simulink library "LibrarySimulinkSASv2016a"  (R2018a)
settings: Solver=ode45  StartTime=0.0  StopTime=10.0  RelTol=1e-3
undefined symbols (not defined in the file or in its folder): IdB, IdL, IdM, shmControl, ts
libraries: LibrarySimulinkSAS x15, $bdroot x1
(part 3 of 4)

== system LibrarySimulinkSASv2016a/V-REP Camera ==
  "Position " : Inport
  Orientation : Inport  Port=2
  Generic Share Memory Block V2.0 : S-Function  FunctionName=matlabGenericSHM  Parameters=nombre,dispositivo,vecE,vecS,tipoE,tipoS,ts  SFunctionDeploymentMode=off  EnableBusSupport=off  mask(Generic Share Memory block V2.0){nombre='vrepCam', dispositivo=id, vecE=[3 3], vecS=[3*ResX*ResY], tipoE=['f' 'f'], tipoS=['C'], ts=-1}
      mask prompts: nombre "Share Memory base name"; dispositivo "Device Number"; vecE "Sizes input vector"; vecS "Sizes output vector"; tipoE "Data Types Inputs"; tipoS "Data Types Outputs"; ts "Sample Time"
      mask help:
      | This block allows Simulink communication with external processes through shared memory. It works on Unix and Windows.
      | More information in Help.
      mask Help:
      | Share Memory base name (nombre): It's the base string of share memory ej 'robot'
      | Device Number (dispositivo): integer that identifies the device between multiple in use
      | Two shared memorys are created with names:
      | "%name_entrada_%dispositivo" for the device input (writing)
      | "%name_salida_%dispositivo" for the device output (reading)
      | Sizes input vector (vecE)  : array that specify the number of inputs (array dimension) and the inputs size (array values)
      | Sizes output vector  (vecS): array that specify the number of outputs (array dimension) and the outputs size (array values)
      | Data types input(tipoE): char array that specify each input types
      | Data types output (tipoS): char array that specify each output types
      | Allowed types are:
      | ('f'=float, 'd'=double, 'i'=int32, 'c'=char, 'C'= unsigned char, 'I'=unsigned int)
      | Example.
      | Suppose the following parameters:
      | nombre='exampleMemory'
      | dispositivo=10
      | vecE=[3 2 1]
      | vecS=[6 5 7 8]
      | tipoE=['f' 'I' 'c']
      | tipoS=['C' 'i' 'd' 'd']
      | 
      | Two Share Memories are created with names:
      | "exampleMemory_entrada_10" (block input)
      | "exampleMemory_salida_10"  (block output)
      | 3 inputs are created:
      |  1° Dimension 3, type "unsigned char"
      |  2° Dimension 2, type "int"
      |  3° Dimension 1, type "char"
      | 4 outputs are created:
      |  1° Dimension 6, type "float"
      |  2° Dimension 5, type "unsigned int"
      |  3° Dimension 7, type "double"
      |  3° Dimension 8, type "double"
      mask Display: image('shmem.jpg')
  toRGB image1 : Subsystem  mask{ResX=ResX, ResY=ResY}
      mask prompts: ResX "Resolucion Camara X"; ResY "Resolucion Camara Y"
      mask Display:
      | port_label('output', 1, 'RGB')
      | port_label('input', 1, 'videoIN')
  RGB : Outport
  charBuff : Outport  Port=2
  wires:
  "Position "/1 -> Generic Share Memory Block V2.0/1
  toRGB image1/1(Out1) -> RGB/1
  Orientation/1 -> Generic Share Memory Block V2.0/2
  Generic Share Memory Block V2.0/1 -> toRGB image1/1(In1), charBuff/1

== system LibrarySimulinkSASv2016a/V-REP Display ==
  "RGB " : Inport
  Generic Shared Memory Block V2 : LibrarySimulinkSAS/Generic Shared Memory Block V2  devInMem='device_actions'  devOutMem='device_sensors'  vecE=[3*ResX*ResY]  vecS=[]  tipoE=['C']  tipoS=[]  ts=ts
  Permute Dimensions : PermuteDimensions  Order=[3,2,1]
  Reshape : Reshape
  toSingle : DataTypeConversion  OutDataTypeStr=uint8
  wires:
  Permute Dimensions/1 -> Reshape/1
  Reshape/1 -> Generic Shared Memory Block V2/1
  "RGB "/1 -> toSingle/1
  toSingle/1 -> Permute Dimensions/1

== system LibrarySimulinkSASv2016a/V-REP Fast Dynamic Joint ==
  jointControl : Inport
  controlType : Inport  Port=2
  PID : Inport  Port=3
  Data Type Conversion : DataTypeConversion
  Data Type Conversion1 : DataTypeConversion
  Data Type Conversion2 : DataTypeConversion
  Generic Shared Memory Block : LibrarySimulinkSAS/Generic Shared Memory Block  nombre='memoriaEjemplo'  dispositivo=10  vecE=[3 2 1]  vecS=[4 5 6 156]  tipoE=['f' 'I' 'c']  tipoS=['C' 'i' 'd' 'd']  ts=0
  cnv : DataTypeConversion
  cnv1 : DataTypeConversion
  cnv2 : DataTypeConversion
  position : Outport
  velocity : Outport  Port=2
  acceleration : Outport  Port=3
  wires:
  Data Type Conversion2/1 -> Generic Shared Memory Block/3
  Data Type Conversion1/1 -> Generic Shared Memory Block/2
  Data Type Conversion/1 -> Generic Shared Memory Block/1
  cnv1/1 -> velocity/1
  cnv/1 -> position/1
  Generic Shared Memory Block/1 -> cnv/1
  Generic Shared Memory Block/2 -> cnv1/1
  controlType/1 -> Data Type Conversion1/1
  jointControl/1 -> Data Type Conversion/1
  cnv2/1 -> acceleration/1
  Generic Shared Memory Block/3 -> cnv2/1
  PID/1 -> Data Type Conversion2/1

== system LibrarySimulinkSASv2016a/V-REP GyroSensor ==
  Data Type Conversion : DataTypeConversion
  Simple Share Memory Block : S-Function  FunctionName=simpleSHM  Parameters=nombre,dispositivo,vecE,vecS,tipoE,tipoS  SFunctionDeploymentMode=off  EnableBusSupport=off  mask(Share Memory Block version 2.0){nombre='VREP_gyroData', dispositivo=n, vecE=[], vecS=[3], tipoE=[], tipoS=['f']}
      mask prompts: nombre "Share Memory base name"; dispositivo "Device Number"; vecE "Sizes input vector"; vecS "Sizes output vector"; tipoE "Data Types Inputs"; tipoS "Data Types Outputs"
      mask help: This block allows Simulink communication with external processes through shared memory. It works on Unix and Windows
      mask Help:
      | Share Memory base name (nombre): It's the base string of share memory ej 'robot'
      | Device Number (dispositivo): integer that identifies the device between multiple in use
      | Only one Share memory will be created, so you should specify only the inputs or outputs but not both
      | One shared memorys is created with name: "%name_%dispositivo"
      | 
      | Sizes input vector (vecE)  : array that specify the number of inputs (array dimension) and the inputs size (array values)
      | Sizes output vector  (vecS): array that specify the number of outputs (array dimension) and the outputs size (array values)
      | Data types input(tipoE): char array that specify each input types
      | Data types output (tipoS): char array that specify each output types
      | Allowed types are:
      | ('f'=float, 'd'=double, 'i'=int32, 'c'=char, 'C'= unsigned char, 'I'=unsigned int)
      | 
      | Example.
      | Suppose the following parameters:
      | nombre='exampleMemory'
      | dispositivo=10
      | vecE=[3 2 1]
      | vecS=[]
      | tipoE=['f' 'I' 'c']
      | tipoS=[]
      | 
      | One Share Memorie is created with name:
      | "exampleMemory_10" (block input)
      | 
      | 3 inputs are created:
      |  1° Dimension 3, type "unsigned char"
      |  2° Dimension 2, type "int"
      |  3° Dimension 1, type "char"
      mask Display: image('memoryChip.png')
  GyroData : Outport
  wires:
  Data Type Conversion/1 -> GyroData/1
  Simple Share Memory Block/1 -> Data Type Conversion/1

== system LibrarySimulinkSASv2016a/V-REP Hokuyo ==
  Generic Shared Memory Block V2.0 : S-Function  FunctionName=matlabGenericSHM  Parameters=nombre,dispositivo,vecE,vecS,tipoE,tipoS,ts  SFunctionDeploymentMode=off  EnableBusSupport=off  mask(Generic Share Memory block V2.0){nombre='vrepHOKUYO', dispositivo=id, vecE=[], vecS=[1 1 1]*683, tipoE=[], tipoS=['f' 'f' 'f'], ts=ts}
      mask prompts: nombre "Share Memory base name"; dispositivo "Device Number"; vecE "Sizes input vector"; vecS "Sizes output vector"; tipoE "Data Types Inputs"; tipoS "Data Types Outputs"; ts "Sample Time"
      mask help:
      | This block allows Simulink communication with external processes through shared memory. It works on Unix and Windows.
      | More information in Help.
      mask Help:
      | Share Memory base name (nombre): It's the base string of share memory ej 'robot'
      | Device Number (dispositivo): integer that identifies the device between multiple in use
      | Two shared memorys are created with names:
      | "%name_entrada_%dispositivo" for the device input (writing)
      | "%name_salida_%dispositivo" for the device output (reading)
      | Sizes input vector (vecE)  : array that specify the number of inputs (array dimension) and the inputs size (array values)
      | Sizes output vector  (vecS): array that specify the number of outputs (array dimension) and the outputs size (array values)
      | Data types input(tipoE): char array that specify each input types
      | Data types output (tipoS): char array that specify each output types
      | Allowed types are:
      | ('f'=float, 'd'=double, 'i'=int32, 'c'=char, 'C'= unsigned char, 'I'=unsigned int)
      | Example.
      | Suppose the following parameters:
      | nombre='exampleMemory'
      | dispositivo=10
      | vecE=[3 2 1]
      | vecS=[6 5 7 8]
      | tipoE=['f' 'I' 'c']
      | tipoS=['C' 'i' 'd' 'd']
      | 
      | Two Share Memories are created with names:
      | "exampleMemory_entrada_10" (block input)
      | "exampleMemory_salida_10"  (block output)
      | 3 inputs are created:
      |  1° Dimension 3, type "unsigned char"
      |  2° Dimension 2, type "int"
      |  3° Dimension 1, type "char"
      | 4 outputs are created:
      |  1° Dimension 6, type "float"
      |  2° Dimension 5, type "unsigned int"
      |  3° Dimension 7, type "double"
      |  3° Dimension 8, type "double"
      mask Display: image('shmem.jpg')
  a doble : DataTypeConversion  OutDataTypeStr=double
  a doble1 : DataTypeConversion  OutDataTypeStr=double
  a doble3 : DataTypeConversion  OutDataTypeStr=double
  ang (rad) : Outport
  ang (deg) : Outport  Port=2
  dist : Outport  Port=3
  wires:
  Generic Shared Memory Block V2.0/3 -> a doble3/1
  Generic Shared Memory Block V2.0/2 -> a doble1/1
  Generic Shared Memory Block V2.0/1 -> a doble/1
  a doble3/1 -> dist/1
  a doble1/1 -> ang (deg)/1
  a doble/1 -> ang (rad)/1

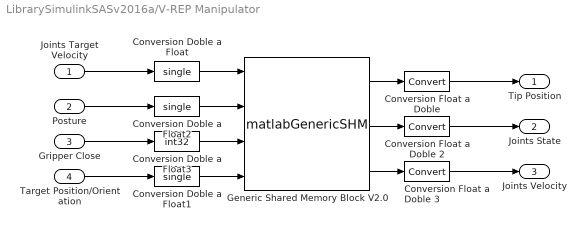
== system LibrarySimulinkSASv2016a/V-REP Manipulator ==
  Joints Target Velocity : Inport
  Posture : Inport  Port=2
  Gripper Close : Inport  Port=3
  Target Position/Orientation : Inport  Port=4
  Conversion Doble a Float : DataTypeConversion  OutDataTypeStr=single
  Conversion Doble a Float1 : DataTypeConversion  OutDataTypeStr=single
  Conversion Doble a Float2 : DataTypeConversion  OutDataTypeStr=single
  Conversion Doble a Float3 : DataTypeConversion  OutDataTypeStr=int32
  "Conversion Float a Doble " : DataTypeConversion
  Conversion Float a Doble 2 : DataTypeConversion
  Conversion Float a Doble 3 : DataTypeConversion
  Generic Shared Memory Block V2.0 : S-Function  FunctionName=matlabGenericSHM  Parameters=nombre,dispositivo,vecE,vecS,tipoE,tipoS,ts  SFunctionDeploymentMode=off  EnableBusSupport=off  mask(Generic Share Memory block V2.0){nombre='RoboArm', dispositivo=id, vecE=[4 4 1 6], vecS=[6 4 4], tipoE=['f' 'f' 'i' 'f'], tipoS=['f' 'f' 'f'], ts=0}
      mask prompts: nombre "Share Memory base name"; dispositivo "Device Number"; vecE "Sizes input vector"; vecS "Sizes output vector"; tipoE "Data Types Inputs"; tipoS "Data Types Outputs"; ts "Sample Time"
      mask help:
      | This block allows Simulink communication with external processes through shared memory. It works on Unix and Windows.
      | More information in Help.
      mask Help:
      | Share Memory base name (nombre): It's the base string of share memory ej 'robot'
      | Device Number (dispositivo): integer that identifies the device between multiple in use
      | Two shared memorys are created with names:
      | "%name_entrada_%dispositivo" for the device input (writing)
      | "%name_salida_%dispositivo" for the device output (reading)
      | Sizes input vector (vecE)  : array that specify the number of inputs (array dimension) and the inputs size (array values)
      | Sizes output vector  (vecS): array that specify the number of outputs (array dimension) and the outputs size (array values)
      | Data types input(tipoE): char array that specify each input types
      | Data types output (tipoS): char array that specify each output types
      | Allowed types are:
      | ('f'=float, 'd'=double, 'i'=int32, 'c'=char, 'C'= unsigned char, 'I'=unsigned int)
      | Example.
      | Suppose the following parameters:
      | nombre='exampleMemory'
      | dispositivo=10
      | vecE=[3 2 1]
      | vecS=[6 5 7 8]
      | tipoE=['f' 'I' 'c']
      | tipoS=['C' 'i' 'd' 'd']
      | 
      | Two Share Memories are created with names:
      | "exampleMemory_entrada_10" (block input)
      | "exampleMemory_salida_10"  (block output)
      | 3 inputs are created:
      |  1° Dimension 3, type "unsigned char"
      |  2° Dimension 2, type "int"
      |  3° Dimension 1, type "char"
      | 4 outputs are created:
      |  1° Dimension 6, type "float"
      |  2° Dimension 5, type "unsigned int"
      |  3° Dimension 7, type "double"
      |  3° Dimension 8, type "double"
      mask Display: image('shmem.jpg')
  Tip Position : Outport
  Joints State : Outport  Port=2
  Joints Velocity : Outport  Port=3
  wires:
  Conversion Doble a Float3/1 -> Generic Shared Memory Block V2.0/3
  Generic Shared Memory Block V2.0/2 -> Conversion Float a Doble 2/1
  Generic Shared Memory Block V2.0/3 -> Conversion Float a Doble 3/1
  Conversion Float a Doble 3/1 -> Joints Velocity/1
  "Conversion Float a Doble "/1 -> Tip Position/1
  Conversion Float a Doble 2/1 -> Joints State/1
  Generic Shared Memory Block V2.0/1 -> "Conversion Float a Doble "/1
  Joints Target Velocity/1 -> Conversion Doble a Float/1
  Gripper Close/1 -> Conversion Doble a Float3/1
  Target Position/Orientation/1 -> Conversion Doble a Float1/1
  Conversion Doble a Float/1 -> Generic Shared Memory Block V2.0/1
  Conversion Doble a Float1/1 -> Generic Shared Memory Block V2.0/4
  Posture/1 -> Conversion Doble a Float2/1
  Conversion Doble a Float2/1 -> Generic Shared Memory Block V2.0/2

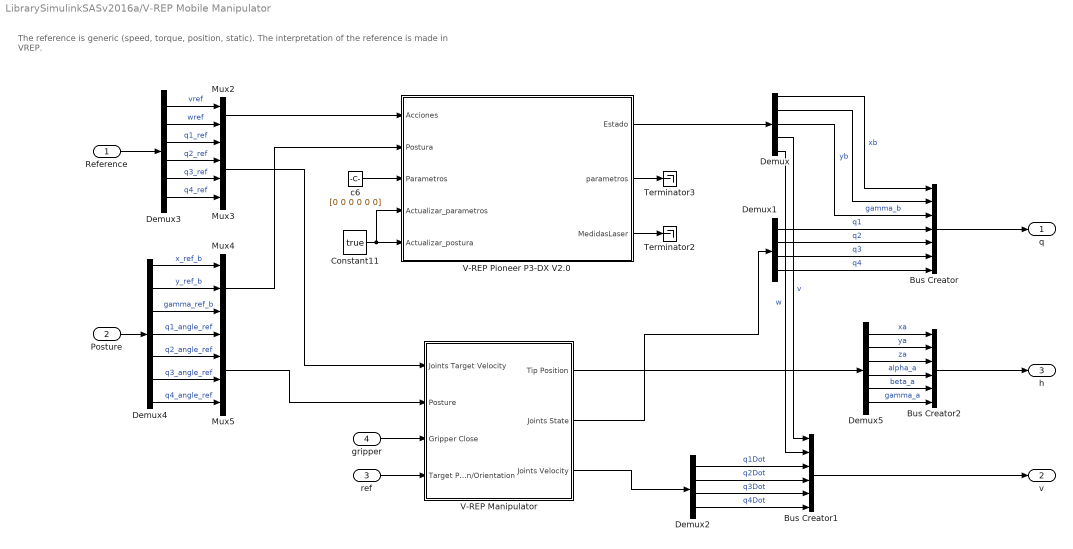
== system LibrarySimulinkSASv2016a/V-REP Mobile Manipulator ==
  note: The reference is generic (speed, torque, position, static). The interpretation of the reference is made in VREP.
  Reference : Inport
  Posture : Inport  Port=2
  ref : Inport  Port=3
  gripper : Inport  Port=4
  Bus Creator : BusCreator  Inputs=7
  Bus Creator1 : BusCreator  Inputs=6
  Bus Creator2 : BusCreator  Inputs=6
  Constant11 : Constant  Value=true
  Demux : Demux  Outputs=5
  Demux1 : Demux
  Demux2 : Demux
  Demux3 : Demux  Outputs=6
  Demux4 : Demux  Outputs=7
  Demux5 : Demux  Outputs=6
  Mux2 : Mux  Inputs=2
  Mux3 : Mux
  Mux4 : Mux  Inputs=3
  Mux5 : Mux
  Terminator2 : Terminator
  Terminator3 : Terminator
  V-REP Manipulator : Subsystem  mask{id=id2}
      mask prompts: id "Id Memoria Compartida"
      mask Display:
      | color('blue');
      | image('phx.jpg')
      | port_label('input', 1, 'Joints Command')
      | port_label('input', 2, 'Posture')
      | port_label('input', 3, 'Gripper Close')
      | port_label('input', 4, 'Target')
      | color('red');
      | port_label('output', 1, 'Tip Position')
      | port_label('output', 2, 'Joints Position')
      | port_label('output', 3, 'Joints Velocity')
  V-REP Pioneer P3-DX V2.0 : Subsystem  mask{id=id1}
      mask prompts: id "Device Number"
      mask Help:
      | External dependencies
      | 
      | Before running the Simulink application initiate the simulation on VREP.
      | 
      | Input Ports
      | 
      | The port 'Actions' transmits control actions to the simulated model. The nature of the control input control type depends on the model set VREP. These inputs may be Forces [N], Speed [m/s; rad/s] or references to the local PID speed controller [m/s; rad/s].
      | Port 'Postura' it is used to load the desired robot posture in the scene.
      | Port 'ActualizarPostura' it is used to set the the posture loded on port 'Postura' in the VREP scene
      | Port 'Parametros' it is use to load the robot internal PID controller
      | Port 'ActualizarParametros' it is used to set the parameters loded in port 'Parametros'
      | 
      | Output Ports
      | 
      | Port 'Estados' contains odometry information in its first three components and the robot linear and angular velocity in its last two components.
      | Port 'Parametros' contains the current parameters set in the robot.
      | Port 'Laser ' contains the Laser dots information.
      | 
      | The Share Memory Estructure is:
      | 
      | typedef struct DEVICE_INPUT {
      | double Acciones[2];
      | double Postura[3];
      | int Parametros[6];
      | bool Actualizar_parametros;
      | bool Actualizar_postura;
      | } VIRTUAL_INPUT;
      | 
      | DEVICE_OUTPUT {
      | double Estado[5];
      | int Parametros[6];
      | unsigned int MedidasLaser[181];
      | } VIRTUAL_OUTPUT;
      mask DialogControl DescGroupVar Prompt: V-REP Simulated Pioneer P3dx
      mask DialogControl DescTextVar Prompt: This block is associated with the VREP model 'vrepModel_PioneerP3DX Hokuyo V2.2.ttm' available from the '\SAS TOOLS\Library V-REP Models & ScenesV1.0.0' folder. More Information on Help button.
      mask DialogControl Control2 Prompt: For multiple devices, set diferent number for each one.
      mask Display:
      | image('pioneer3dx_virtual.png')
      | color('red')
      | port_label('input',1,'acciones')
      | port_label('input',2,'postura')
      | port_label('input',3,'parametros')
      | port_label('input',4,'parametrosSend')
      | port_label('input',5,'posturaSend')
      | color('white')
      | port_label('output',1,'estado')
      | port_label('output',2,'parametros')
      | port_label('output',3,'laser')
  c6 : Constant  Value=[0 0 0 0 0 0]  OutDataTypeStr=int32
  q : Outport
  v : Outport  Port=2
  h : Outport  Port=3
  wires:
  Demux5/6 -> Bus Creator2/6  "gamma_a"
  Demux5/5 -> Bus Creator2/5  "beta_a"
  Demux5/4 -> Bus Creator2/4  "alpha_a"
  Demux5/3 -> Bus Creator2/3  "za"
  Demux5/2 -> Bus Creator2/2  "ya"
  Demux5/1 -> Bus Creator2/1  "xa"
  Demux1/4 -> Bus Creator/7  "q4"
  Demux/4 -> Bus Creator1/1  "v"
  Demux/5 -> Bus Creator1/2  "w"
  V-REP Manipulator/2(Joints State) -> Demux1/1
  Demux2/4 -> Bus Creator1/6  "q4Dot"
  V-REP Manipulator/3(Joints Velocity) -> Demux2/1
  Demux2/3 -> Bus Creator1/5  "q3Dot"
  Demux2/2 -> Bus Creator1/4  "q2Dot"
  Demux2/1 -> Bus Creator1/3  "q1Dot"
  Reference/1 -> Demux3/1
  ref/1 -> V-REP Manipulator/4(Target Position/Orientation)
  Mux4/1 -> V-REP Pioneer P3-DX V2.0/2(Postura)
  c6/1 -> V-REP Pioneer P3-DX V2.0/3(Parametros)
  Constant11/1 -> V-REP Pioneer P3-DX V2.0/5(Actualizar_postura), V-REP Pioneer P3-DX V2.0/4(Actualizar_parametros)
  V-REP Pioneer P3-DX V2.0/3(MedidasLaser) -> Terminator2/1
  V-REP Pioneer P3-DX V2.0/2(parametros) -> Terminator3/1
  Mux2/1 -> V-REP Pioneer P3-DX V2.0/1(Acciones)
  Demux1/3 -> Bus Creator/6  "q3"
  Demux1/2 -> Bus Creator/5  "q2"
  Demux1/1 -> Bus Creator/4  "q1"
  Demux/1 -> Bus Creator/1  "xb"
  Demux/2 -> Bus Creator/2  "yb"
  Demux/3 -> Bus Creator/3  "gamma_b"
  V-REP Pioneer P3-DX V2.0/1(Estado) -> Demux/1
  V-REP Manipulator/1(Tip Position) -> Demux5/1
  Bus Creator/1 -> q/1
  Bus Creator2/1 -> h/1
  Bus Creator1/1 -> v/1
  Demux3/1 -> Mux2/1  "vref"
  Demux3/2 -> Mux2/2  "wref"
  Demux3/3 -> Mux3/1  "q1_ref"
  Demux3/4 -> Mux3/2  "q2_ref"
  Demux3/5 -> Mux3/3  "q3_ref"
  Demux3/6 -> Mux3/4  "q4_ref"
  Demux4/1 -> Mux4/1  "x_ref_b"
  Demux4/2 -> Mux4/2  "y_ref_b"
  Demux4/3 -> Mux4/3  "gamma_ref_b"
  Demux4/4 -> Mux5/1  "q1_angle_ref"
  Demux4/5 -> Mux5/2  "q2_angle_ref"
  Demux4/6 -> Mux5/3  "q3_angle_ref"
  Demux4/7 -> Mux5/4  "q4_angle_ref"
  Posture/1 -> Demux4/1
  Mux5/1 -> V-REP Manipulator/2(Posture)
  gripper/1 -> V-REP Manipulator/3(Gripper Close)
  Mux3/1 -> V-REP Manipulator/1(Joints Target Velocity)

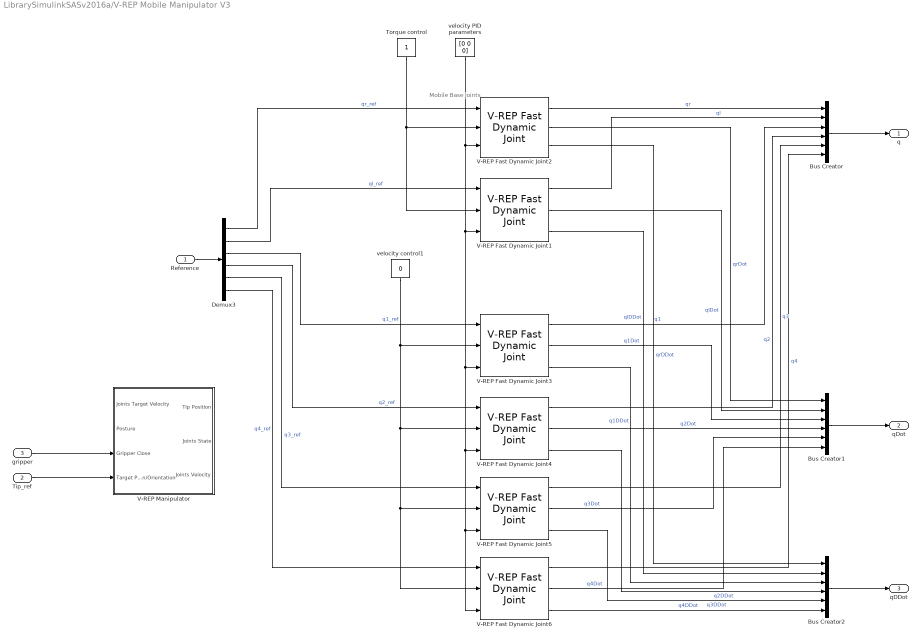
== system LibrarySimulinkSASv2016a/V-REP Mobile Manipulator V3 ==
  note: Mobile Base Joints
  Reference : Inport
  Tip_ref : Inport  Port=2
  gripper : Inport  Port=3
  Bus Creator : BusCreator  Inputs=6
  Bus Creator1 : BusCreator  Inputs=6
  Bus Creator2 : BusCreator  Inputs=6
  Demux3 : Demux  Outputs=6
  Torque control : Constant
  V-REP Fast Dynamic Joint{1..6} (6 alike) : LibrarySimulinkSAS/V-REP Fast Dynamic Joint  id=1
  V-REP Manipulator : Subsystem  mask{id=id+30}
      mask prompts: id "Id Memoria Compartida"
      mask Display:
      | color('blue');
      | image('phx.jpg')
      | port_label('input', 1, 'Joints Command')
      | port_label('input', 2, 'Posture')
      | port_label('input', 3, 'Gripper Close')
      | port_label('input', 4, 'Target')
      | color('red');
      | port_label('output', 1, 'Tip Position')
      | port_label('output', 2, 'Joints Position')
      | port_label('output', 3, 'Joints Velocity')
  velocity PID parameters : Constant  Value=[0 0 0]
  velocity control1 : Constant  Value=0
  q : Outport
  qDot : Outport  Port=2
  qDDot : Outport  Port=3
  wires:
  gripper/1 -> V-REP Manipulator/3(Gripper Close)
  Demux3/6 -> V-REP Fast Dynamic Joint6/1  "q4_ref"
  Demux3/5 -> V-REP Fast Dynamic Joint5/1  "q3_ref"
  Demux3/4 -> V-REP Fast Dynamic Joint4/1  "q2_ref"
  Demux3/3 -> V-REP Fast Dynamic Joint3/1  "q1_ref"
  Bus Creator/1 -> q/1
  V-REP Fast Dynamic Joint1/1 -> Bus Creator/2  "ql"
  V-REP Fast Dynamic Joint2/1 -> Bus Creator/1  "qr"
  V-REP Fast Dynamic Joint3/1 -> Bus Creator/3  "q1"
  V-REP Fast Dynamic Joint4/1 -> Bus Creator/4  "q2"
  V-REP Fast Dynamic Joint5/1 -> Bus Creator/5  "q3"
  Tip_ref/1 -> V-REP Manipulator/4(Target Position/Orientation)
  Reference/1 -> Demux3/1
  V-REP Fast Dynamic Joint6/1 -> Bus Creator/6  "q4"
  Torque control/1 -> V-REP Fast Dynamic Joint1/2, V-REP Fast Dynamic Joint2/2
  velocity PID parameters/1 -> V-REP Fast Dynamic Joint3/3, V-REP Fast Dynamic Joint4/3, V-REP Fast Dynamic Joint5/3, V-REP Fast Dynamic Joint6/3, V-REP Fast Dynamic Joint1/3, V-REP Fast Dynamic Joint2/3
  Bus Creator1/1 -> qDot/1
  Demux3/1 -> V-REP Fast Dynamic Joint2/1  "qr_ref"
  Demux3/2 -> V-REP Fast Dynamic Joint1/1  "ql_ref"
  V-REP Fast Dynamic Joint2/2 -> Bus Creator1/1  "qrDot"
  V-REP Fast Dynamic Joint1/2 -> Bus Creator1/2  "qlDot"
  V-REP Fast Dynamic Joint3/2 -> Bus Creator1/3  "q1Dot"
  V-REP Fast Dynamic Joint4/2 -> Bus Creator1/4  "q2Dot"
  V-REP Fast Dynamic Joint5/2 -> Bus Creator1/5  "q3Dot"
  V-REP Fast Dynamic Joint6/2 -> Bus Creator1/6  "q4Dot"
  Bus Creator2/1 -> qDDot/1
  V-REP Fast Dynamic Joint2/3 -> Bus Creator2/1  "qrDDot"
  V-REP Fast Dynamic Joint1/3 -> Bus Creator2/2  "qlDDot"
  V-REP Fast Dynamic Joint3/3 -> Bus Creator2/3  "q1DDot"
  V-REP Fast Dynamic Joint4/3 -> Bus Creator2/4  "q2DDot"
  V-REP Fast Dynamic Joint5/3 -> Bus Creator2/5  "q3DDot"
  V-REP Fast Dynamic Joint6/3 -> Bus Creator2/6  "q4DDot"
  velocity control1/1 -> V-REP Fast Dynamic Joint3/2, V-REP Fast Dynamic Joint4/2, V-REP Fast Dynamic Joint5/2, V-REP Fast Dynamic Joint6/2

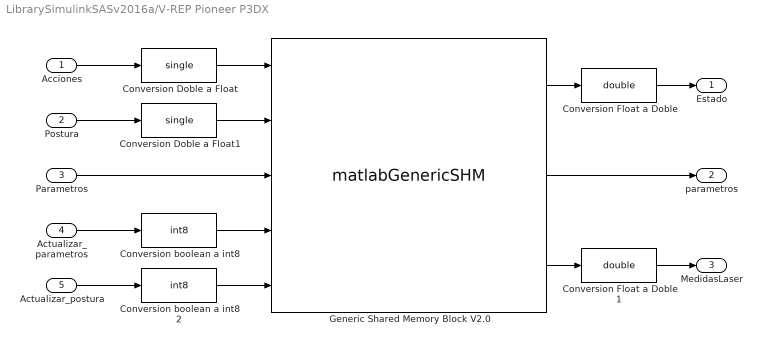
== system LibrarySimulinkSASv2016a/V-REP Pioneer P3DX ==
  Acciones : Inport
  Postura : Inport  Port=2
  Parametros : Inport  Port=3
  Actualizar_parametros : Inport  Port=4
  Actualizar_postura : Inport  Port=5
  Conversion Doble a Float : DataTypeConversion  OutDataTypeStr=single
  Conversion Doble a Float1 : DataTypeConversion  OutDataTypeStr=single
  "Conversion Float a Doble " : DataTypeConversion  OutDataTypeStr=double
  Conversion Float a Doble 1 : DataTypeConversion  OutDataTypeStr=double
  Conversion boolean a int8 : DataTypeConversion  OutDataTypeStr=int8
  Conversion boolean a int8 2 : DataTypeConversion  OutDataTypeStr=int8
  Generic Shared Memory Block V2.0 : S-Function  FunctionName=matlabGenericSHM  Parameters=nombre,dispositivo,vecE,vecS,tipoE,tipoS,ts  SFunctionDeploymentMode=off  EnableBusSupport=off  mask(Generic Share Memory block V2.0){nombre='VREP_PIONEER', dispositivo=id, vecE=[2 3 6 1 1], vecS=[5 6 181], tipoE=['f' 'f' 'i' 'c' 'c'], tipoS=['f' 'i' 'I'], ts=0}
      mask prompts: nombre "Share Memory base name"; dispositivo "Device Number"; vecE "Sizes input vector"; vecS "Sizes output vector"; tipoE "Data Types Inputs"; tipoS "Data Types Outputs"; ts "Sample Time"
      mask help:
      | This block allows Simulink communication with external processes through shared memory. It works on Unix and Windows.
      | More information in Help.
      mask Help:
      | Share Memory base name (nombre): It's the base string of share memory ej 'robot'
      | Device Number (dispositivo): integer that identifies the device between multiple in use
      | Two shared memorys are created with names:
      | "%name_entrada_%dispositivo" for the device input (writing)
      | "%name_salida_%dispositivo" for the device output (reading)
      | Sizes input vector (vecE)  : array that specify the number of inputs (array dimension) and the inputs size (array values)
      | Sizes output vector  (vecS): array that specify the number of outputs (array dimension) and the outputs size (array values)
      | Data types input(tipoE): char array that specify each input types
      | Data types output (tipoS): char array that specify each output types
      | Allowed types are:
      | ('f'=float, 'd'=double, 'i'=int32, 'c'=char, 'C'= unsigned char, 'I'=unsigned int)
      | Example.
      | Suppose the following parameters:
      | nombre='exampleMemory'
      | dispositivo=10
      | vecE=[3 2 1]
      | vecS=[6 5 7 8]
      | tipoE=['f' 'I' 'c']
      | tipoS=['C' 'i' 'd' 'd']
      | 
      | Two Share Memories are created with names:
      | "exampleMemory_entrada_10" (block input)
      | "exampleMemory_salida_10"  (block output)
      | 3 inputs are created:
      |  1° Dimension 3, type "unsigned char"
      |  2° Dimension 2, type "int"
      |  3° Dimension 1, type "char"
      | 4 outputs are created:
      |  1° Dimension 6, type "float"
      |  2° Dimension 5, type "unsigned int"
      |  3° Dimension 7, type "double"
      |  3° Dimension 8, type "double"
      mask Display: image('shmem.jpg')
  Estado : Outport
  parametros : Outport  Port=2
  MedidasLaser : Outport  Port=3
  wires:
  Conversion Doble a Float1/1 -> Generic Shared Memory Block V2.0/2
  Generic Shared Memory Block V2.0/1 -> "Conversion Float a Doble "/1
  Actualizar_postura/1 -> Conversion boolean a int8 2/1
  Postura/1 -> Conversion Doble a Float1/1
  Actualizar_parametros/1 -> Conversion boolean a int8/1
  Acciones/1 -> Conversion Doble a Float/1
  "Conversion Float a Doble "/1 -> Estado/1
  Parametros/1 -> Generic Shared Memory Block V2.0/3
  Conversion boolean a int8/1 -> Generic Shared Memory Block V2.0/4
  Conversion boolean a int8 2/1 -> Generic Shared Memory Block V2.0/5
  Conversion Doble a Float/1 -> Generic Shared Memory Block V2.0/1
  Generic Shared Memory Block V2.0/2 -> parametros/1
  Generic Shared Memory Block V2.0/3 -> Conversion Float a Doble 1/1
  Conversion Float a Doble 1/1 -> MedidasLaser/1

== system LibrarySimulinkSASv2016a/V-REP Pioneer STATIC ==
  In1 : Inport
  In2 : Inport  Port=2
  Data Type Conversion : DataTypeConversion  OutDataTypeStr=single
  Data Type Conversion1 : DataTypeConversion  OutDataTypeStr=double
  Data Type Conversion2 : DataTypeConversion  OutDataTypeStr=single
  Data Type Conversion3 : DataTypeConversion  OutDataTypeStr=double
  Generic Shared Memory Block V2.0 : S-Function  FunctionName=matlabGenericSHM  Parameters=nombre,dispositivo,vecE,vecS,tipoE,tipoS  SFunctionDeploymentMode=off  EnableBusSupport=off  mask(Generic Share Memory block V2.0){nombre='PioneerEstatico', dispositivo=id, vecE=[3 1], vecS=[3 1], tipoE=['f' 'f'], tipoS=['f' 'f']}
      mask prompts: nombre "Share Memory base name"; dispositivo "Device Number"; vecE "Sizes input vector"; vecS "Sizes output vector"; tipoE "Data Types Inputs"; tipoS "Data Types Outputs"
      mask help:
      | This block allows Simulink communication with external processes through shared memory. It works on Unix and Windows.
      | More information in Help.
      mask Help:
      | Share Memory base name (nombre): It's the base string of share memory ej 'robot'
      | Device Number (dispositivo): integer that identifies the device between multiple in use
      | Two shared memorys are created with names:
      | "%name_entrada_%dispositivo" for the device input (writing)
      | "%name_salida_%dispositivo" for the device output (reading)
      | Sizes input vector (vecE)  : array that specify the number of inputs (array dimension) and the inputs size (array values)
      | Sizes output vector  (vecS): array that specify the number of outputs (array dimension) and the outputs size (array values)
      | Data types input(tipoE): char array that specify each input types
      | Data types output (tipoS): char array that specify each output types
      | Allowed types are:
      | ('f'=float, 'd'=double, 'i'=int32, 'c'=char, 'C'= unsigned char, 'I'=unsigned int)
      | Example.
      | Suppose the following parameters:
      | nombre='exampleMemory'
      | dispositivo=10
      | vecE=[3 2 1]
      | vecS=[6 5 7 8]
      | tipoE=['f' 'I' 'c']
      | tipoS=['C' 'i' 'd' 'd']
      | 
      | Two Share Memories are created with names:
      | "exampleMemory_entrada_10" (block input)
      | "exampleMemory_salida_10"  (block output)
      | 3 inputs are created:
      |  1° Dimension 3, type "unsigned char"
      |  2° Dimension 2, type "int"
      |  3° Dimension 1, type "char"
      | 4 outputs are created:
      |  1° Dimension 6, type "float"
      |  2° Dimension 5, type "unsigned int"
      |  3° Dimension 7, type "double"
      |  3° Dimension 8, type "double"
      mask Display: image('shmem.jpg')
  Out1 : Outport
  Out2 : Outport  Port=2
  wires:
  In1/1 -> Data Type Conversion/1
  Data Type Conversion/1 -> Generic Shared Memory Block V2.0/1
  Generic Shared Memory Block V2.0/1 -> Data Type Conversion1/1
  Data Type Conversion1/1 -> Out1/1
  Data Type Conversion2/1 -> Generic Shared Memory Block V2.0/2
  In2/1 -> Data Type Conversion2/1
  Data Type Conversion3/1 -> Out2/1
  Generic Shared Memory Block V2.0/2 -> Data Type Conversion3/1

== system LibrarySimulinkSASv2016a/V-REP static Quadricopter ==
  Position_IN : Inport
  Orientation_IN : Inport  Port=2
  Data Type Conversion{,1..5} (6 alike) : DataTypeConversion
  Generic Shared Memory Block : S-Function  FunctionName=matlabGenericSHM  Parameters=nombre,dispositivo,vecE,vecS,tipoE,tipoS  SFunctionDeploymentMode=off  EnableBusSupport=off  mask(Generic Share Memory block V2.0){nombre='static_Object', dispositivo=Id, vecE=[3 3 ], vecS=[3 3 3 3], tipoE=['f' 'f'], tipoS=['f' 'f' 'f' 'f']}
      mask prompts: nombre "Share Memory base name"; dispositivo "Device Number"; vecE "Sizes input vector"; vecS "Sizes output vector"; tipoE "Data Types Inputs"; tipoS "Data Types Outputs"
      mask help:
      | This block allows Simulink communication with external processes through shared memory. It works on Unix and Windows.
      | More information in Help.
      mask Help:
      | Share Memory base name (nombre): It's the base string of share memory ej 'robot'
      | Device Number (dispositivo): integer that identifies the device between multiple in use
      | Two shared memorys are created with names:
      | "%name_entrada_%dispositivo" for the device input (writing)
      | "%name_salida_%dispositivo" for the device output (reading)
      | Sizes input vector (vecE)  : array that specify the number of inputs (array dimension) and the inputs size (array values)
      | Sizes output vector  (vecS): array that specify the number of outputs (array dimension) and the outputs size (array values)
      | Data types input(tipoE): char array that specify each input types
      | Data types output (tipoS): char array that specify each output types
      | Allowed types are:
      | ('f'=float, 'd'=double, 'i'=int32, 'c'=char, 'C'= unsigned char, 'I'=unsigned int)
      | Example.
      | Suppose the following parameters:
      | nombre='exampleMemory'
      | dispositivo=10
      | vecE=[3 2 1]
      | vecS=[6 5 7 8]
      | tipoE=['f' 'I' 'c']
      | tipoS=['C' 'i' 'd' 'd']
      | 
      | Two Share Memories are created with names:
      | "exampleMemory_entrada_10" (block input)
      | "exampleMemory_salida_10"  (block output)
      | 3 inputs are created:
      |  1° Dimension 3, type "unsigned char"
      |  2° Dimension 2, type "int"
      |  3° Dimension 1, type "char"
      | 4 outputs are created:
      |  1° Dimension 6, type "float"
      |  2° Dimension 5, type "unsigned int"
      |  3° Dimension 7, type "double"
      |  3° Dimension 8, type "double"
      mask Display: image('shmem.jpg')
  Position : Outport
  Orientation : Outport  Port=2
  Linear Velocity : Outport  Port=3
  Angular Velocity : Outport  Port=4
  wires:
  Position_IN/1 -> Data Type Conversion/1
  Orientation_IN/1 -> Data Type Conversion1/1
  Generic Shared Memory Block/1 -> Data Type Conversion2/1
  Generic Shared Memory Block/2 -> Data Type Conversion3/1
  Data Type Conversion2/1 -> Position/1
  Data Type Conversion1/1 -> Generic Shared Memory Block/2
  Data Type Conversion/1 -> Generic Shared Memory Block/1
  Data Type Conversion3/1 -> Orientation/1
  Data Type Conversion4/1 -> Linear Velocity/1
  Data Type Conversion5/1 -> Angular Velocity/1
  Generic Shared Memory Block/3 -> Data Type Conversion4/1
  Generic Shared Memory Block/4 -> Data Type Conversion5/1

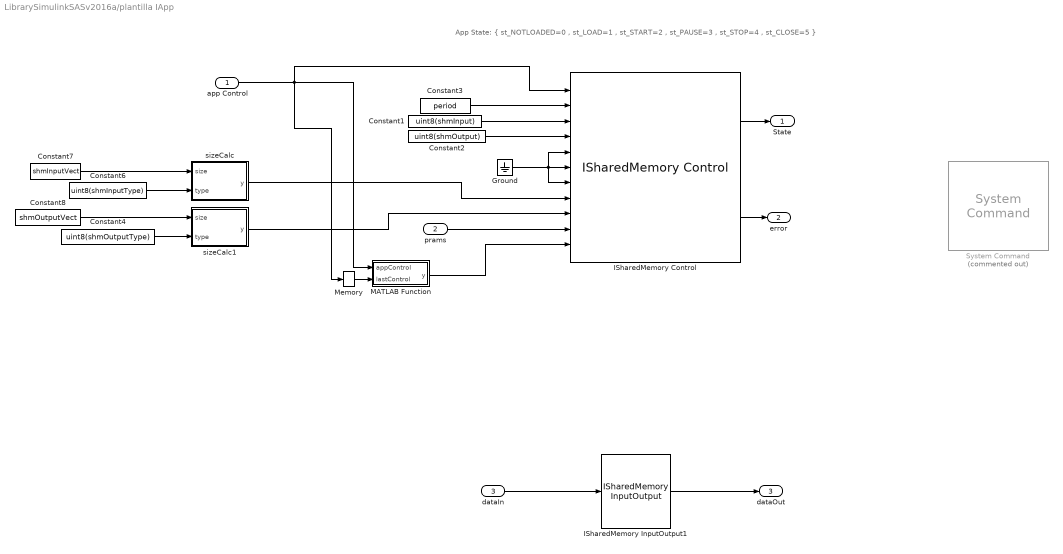
== system LibrarySimulinkSASv2016a/plantilla IApp ==
  note: App State: { st_NOTLOADED=0 , st_LOAD=1 , st_START=2 , st_PAUSE=3 , st_STOP=4 , st_CLOSE=5 }
  app Control : Inport
  prams : Inport  Port=2
  dataIn : Inport  Port=3
  Constant1 : Constant  Value=uint8(shmInput)
  Constant2 : Constant  Value=uint8(shmOutput)
  Constant3 : Constant  Value=period
  Constant4 : Constant  Value=uint8(shmOutputType)  OutDataTypeStr=uint8
  Constant6 : Constant  Value=uint8(shmInputType)  OutDataTypeStr=uint8
  Constant7 : Constant  Value=shmInputVect
  Constant8 : Constant  Value=shmOutputVect
  Ground : Ground
  ISharedMemory Control : LibrarySimulinkSAS/ISharedMemory Control  shmControl=shmControl
  ISharedMemory InputOutput1 : LibrarySimulinkSAS/ISharedMemory InputOutput  nameIn=shmInput  nameOut=shmOutput  vecE=shmInputVect  vecS=shmOutputVect  tipoE=shmInputType  tipoS=shmOutputType  inOff=shmInputOffset  outOff=shmOutputOffset  ts=-1  trigg=off
  MATLAB Function : MATLAB Function  TreatAsAtomicUnit=on
  Memory : Memory  InitialCondition=25
  System Command : LibrarySimulinkSAS/System Command  Priority=0  Commented=on  start=' start SASDelay shmcontrol & timeout /t 10'  update=''  outputs=''  terminate='taskkill /IM SASDelay.exe'
  sizeCalc : MATLAB Function  TreatAsAtomicUnit=on
  sizeCalc1 : MATLAB Function  TreatAsAtomicUnit=on
  State : Outport
  error : Outport  Port=2
  dataOut : Outport  Port=3
  wires:
  MATLAB Function/1(y) -> ISharedMemory Control/11
  Constant2/1 -> ISharedMemory Control/4
  Constant1/1 -> ISharedMemory Control/3
  ISharedMemory Control/2 -> error/1
  ISharedMemory Control/1 -> State/1
  app Control/1 -> ISharedMemory Control/1, MATLAB Function/1(appControl), Memory/1
  Constant3/1 -> ISharedMemory Control/2
  Memory/1 -> MATLAB Function/2(lastControl)
  sizeCalc1/1(y) -> ISharedMemory Control/9
  prams/1 -> ISharedMemory Control/10
  sizeCalc/1(y) -> ISharedMemory Control/8
  Constant7/1 -> sizeCalc/1(size)
  Constant6/1 -> sizeCalc/2(type)
  Constant8/1 -> sizeCalc1/1(size)
  Constant4/1 -> sizeCalc1/2(type)
  dataIn/1 -> ISharedMemory InputOutput1/1
  ISharedMemory InputOutput1/1 -> dataOut/1
  Ground/1 -> ISharedMemory Control/7, ISharedMemory Control/5, ISharedMemory Control/6

== system LibrarySimulinkSASv2016a/system ==
  In1 : Inport
  In2 : Inport  Port=2
  ISharedMemory InputOutput : $bdroot/ISharedMemory InputOutput  nameIn=strcat(nombre,'_entrada_',int2str(dispositivo))  nameOut=strcat(nombre,'_salida_',int2str(dispositivo))  vecE=vecE  vecS=vecS  tipoE=tipoE  tipoS=tipoS  inOff=0  outOff=0  ts=ts  trigg=off
  Out1 : Outport
  Out2 : Outport  Port=2
  wires:
  ISharedMemory InputOutput/2 -> Out2/1
  In1/1 -> ISharedMemory InputOutput/1
  In2/1 -> ISharedMemory InputOutput/2
  ISharedMemory InputOutput/1 -> Out1/1

== system LibrarySimulinkSASv2016a/Ardrone Parrot/toRGB image1 ==
  In1 : Inport
  Permute Dimensions : PermuteDimensions  Order=[3 2 1]
  Reshape : Reshape  OutputDimensionality=Customize  OutputDimensions=[3,ResX,ResY]
  Out1 : Outport
  wires:
  Reshape/1 -> Permute Dimensions/1
  In1/1 -> Reshape/1
  Permute Dimensions/1 -> Out1/1

== system LibrarySimulinkSASv2016a/V-REP Camera/toRGB image1 ==
  In1 : Inport
  Permute Dimensions : PermuteDimensions  Order=[3 2 1]
  Reshape : Reshape  OutputDimensionality=Customize  OutputDimensions=[3,ResX,ResY]
  Out1 : Outport
  wires:
  In1/1 -> Reshape/1
  Reshape/1 -> Permute Dimensions/1
  Permute Dimensions/1 -> Out1/1

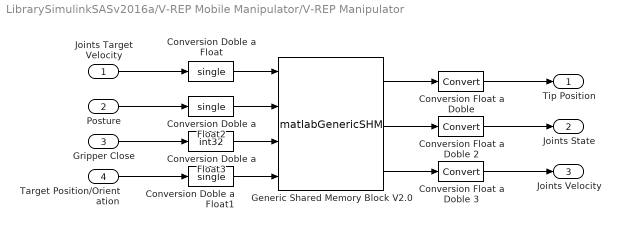
== system LibrarySimulinkSASv2016a/V-REP Mobile Manipulator/V-REP Manipulator ==
  Joints Target Velocity : Inport
  Posture : Inport  Port=2
  Gripper Close : Inport  Port=3
  Target Position/Orientation : Inport  Port=4
  Conversion Doble a Float : DataTypeConversion  OutDataTypeStr=single
  Conversion Doble a Float1 : DataTypeConversion  OutDataTypeStr=single
  Conversion Doble a Float2 : DataTypeConversion  OutDataTypeStr=single
  Conversion Doble a Float3 : DataTypeConversion  OutDataTypeStr=int32
  "Conversion Float a Doble " : DataTypeConversion
  Conversion Float a Doble 2 : DataTypeConversion
  Conversion Float a Doble 3 : DataTypeConversion
  Generic Shared Memory Block V2.0 : S-Function  FunctionName=matlabGenericSHM  Parameters=nombre,dispositivo,vecE,vecS,tipoE,tipoS  SFunctionDeploymentMode=off  EnableBusSupport=off  mask(Generic Share Memory block V2.0){nombre='RoboArm', dispositivo=id, vecE=[4 4 1 6], vecS=[6 4 4], tipoE=['f' 'f' 'i' 'f'], tipoS=['f' 'f' 'f']}
      mask prompts: nombre "Share Memory base name"; dispositivo "Device Number"; vecE "Sizes input vector"; vecS "Sizes output vector"; tipoE "Data Types Inputs"; tipoS "Data Types Outputs"
      mask help:
      | This block allows Simulink communication with external processes through shared memory. It works on Unix and Windows.
      | More information in Help.
      mask Help:
      | Share Memory base name (nombre): It's the base string of share memory ej 'robot'
      | Device Number (dispositivo): integer that identifies the device between multiple in use
      | Two shared memorys are created with names:
      | "%name_entrada_%dispositivo" for the device input (writing)
      | "%name_salida_%dispositivo" for the device output (reading)
      | Sizes input vector (vecE)  : array that specify the number of inputs (array dimension) and the inputs size (array values)
      | Sizes output vector  (vecS): array that specify the number of outputs (array dimension) and the outputs size (array values)
      | Data types input(tipoE): char array that specify each input types
      | Data types output (tipoS): char array that specify each output types
      | Allowed types are:
      | ('f'=float, 'd'=double, 'i'=int32, 'c'=char, 'C'= unsigned char, 'I'=unsigned int)
      | Example.
      | Suppose the following parameters:
      | nombre='exampleMemory'
      | dispositivo=10
      | vecE=[3 2 1]
      | vecS=[6 5 7 8]
      | tipoE=['f' 'I' 'c']
      | tipoS=['C' 'i' 'd' 'd']
      | 
      | Two Share Memories are created with names:
      | "exampleMemory_entrada_10" (block input)
      | "exampleMemory_salida_10"  (block output)
      | 3 inputs are created:
      |  1° Dimension 3, type "unsigned char"
      |  2° Dimension 2, type "int"
      |  3° Dimension 1, type "char"
      | 4 outputs are created:
      |  1° Dimension 6, type "float"
      |  2° Dimension 5, type "unsigned int"
      |  3° Dimension 7, type "double"
      |  3° Dimension 8, type "double"
      mask Display: image('shmem.jpg')
  Tip Position : Outport
  Joints State : Outport  Port=2
  Joints Velocity : Outport  Port=3
  wires:
  Conversion Doble a Float2/1 -> Generic Shared Memory Block V2.0/2
  Posture/1 -> Conversion Doble a Float2/1
  Conversion Doble a Float1/1 -> Generic Shared Memory Block V2.0/4
  Conversion Doble a Float/1 -> Generic Shared Memory Block V2.0/1
  Target Position/Orientation/1 -> Conversion Doble a Float1/1
  Gripper Close/1 -> Conversion Doble a Float3/1
  Joints Target Velocity/1 -> Conversion Doble a Float/1
  Generic Shared Memory Block V2.0/1 -> "Conversion Float a Doble "/1
  Conversion Float a Doble 2/1 -> Joints State/1
  "Conversion Float a Doble "/1 -> Tip Position/1
  Conversion Float a Doble 3/1 -> Joints Velocity/1
  Generic Shared Memory Block V2.0/3 -> Conversion Float a Doble 3/1
  Generic Shared Memory Block V2.0/2 -> Conversion Float a Doble 2/1
  Conversion Doble a Float3/1 -> Generic Shared Memory Block V2.0/3

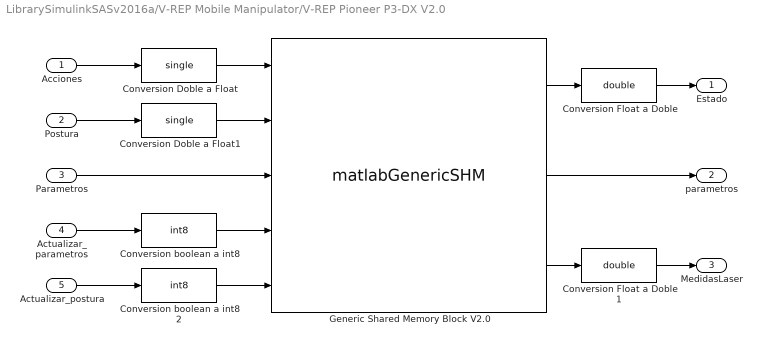
== system LibrarySimulinkSASv2016a/V-REP Mobile Manipulator/V-REP Pioneer P3-DX V2.0 ==
  Acciones : Inport
  Postura : Inport  Port=2
  Parametros : Inport  Port=3
  Actualizar_parametros : Inport  Port=4
  Actualizar_postura : Inport  Port=5
  Conversion Doble a Float : DataTypeConversion  OutDataTypeStr=single
  Conversion Doble a Float1 : DataTypeConversion  OutDataTypeStr=single
  "Conversion Float a Doble " : DataTypeConversion  OutDataTypeStr=double
  Conversion Float a Doble 1 : DataTypeConversion  OutDataTypeStr=double
  Conversion boolean a int8 : DataTypeConversion  OutDataTypeStr=int8
  Conversion boolean a int8 2 : DataTypeConversion  OutDataTypeStr=int8
  Generic Shared Memory Block V2.0 : S-Function  FunctionName=matlabGenericSHM  Parameters=nombre,dispositivo,vecE,vecS,tipoE,tipoS  SFunctionDeploymentMode=off  EnableBusSupport=off  mask(Generic Share Memory block V2.0){nombre='VREP_PIONEER', dispositivo=id, vecE=[2 3 6 1 1], vecS=[5 6 181], tipoE=['f' 'f' 'i' 'c' 'c'], tipoS=['f' 'i' 'I']}
      mask prompts: nombre "Share Memory base name"; dispositivo "Device Number"; vecE "Sizes input vector"; vecS "Sizes output vector"; tipoE "Data Types Inputs"; tipoS "Data Types Outputs"
      mask help:
      | This block allows Simulink communication with external processes through shared memory. It works on Unix and Windows.
      | More information in Help.
      mask Help:
      | Share Memory base name (nombre): It's the base string of share memory ej 'robot'
      | Device Number (dispositivo): integer that identifies the device between multiple in use
      | Two shared memorys are created with names:
      | "%name_entrada_%dispositivo" for the device input (writing)
      | "%name_salida_%dispositivo" for the device output (reading)
      | Sizes input vector (vecE)  : array that specify the number of inputs (array dimension) and the inputs size (array values)
      | Sizes output vector  (vecS): array that specify the number of outputs (array dimension) and the outputs size (array values)
      | Data types input(tipoE): char array that specify each input types
      | Data types output (tipoS): char array that specify each output types
      | Allowed types are:
      | ('f'=float, 'd'=double, 'i'=int32, 'c'=char, 'C'= unsigned char, 'I'=unsigned int)
      | Example.
      | Suppose the following parameters:
      | nombre='exampleMemory'
      | dispositivo=10
      | vecE=[3 2 1]
      | vecS=[6 5 7 8]
      | tipoE=['f' 'I' 'c']
      | tipoS=['C' 'i' 'd' 'd']
      | 
      | Two Share Memories are created with names:
      | "exampleMemory_entrada_10" (block input)
      | "exampleMemory_salida_10"  (block output)
      | 3 inputs are created:
      |  1° Dimension 3, type "unsigned char"
      |  2° Dimension 2, type "int"
      |  3° Dimension 1, type "char"
      | 4 outputs are created:
      |  1° Dimension 6, type "float"
      |  2° Dimension 5, type "unsigned int"
      |  3° Dimension 7, type "double"
      |  3° Dimension 8, type "double"
      mask Display: image('shmem.jpg')
  Estado : Outport
  parametros : Outport  Port=2
  MedidasLaser : Outport  Port=3
  wires:
  Conversion Float a Doble 1/1 -> MedidasLaser/1
  Generic Shared Memory Block V2.0/3 -> Conversion Float a Doble 1/1
  Generic Shared Memory Block V2.0/2 -> parametros/1
  Conversion Doble a Float/1 -> Generic Shared Memory Block V2.0/1
  Conversion boolean a int8 2/1 -> Generic Shared Memory Block V2.0/5
  Conversion boolean a int8/1 -> Generic Shared Memory Block V2.0/4
  Parametros/1 -> Generic Shared Memory Block V2.0/3
  "Conversion Float a Doble "/1 -> Estado/1
  Acciones/1 -> Conversion Doble a Float/1
  Actualizar_parametros/1 -> Conversion boolean a int8/1
  Postura/1 -> Conversion Doble a Float1/1
  Actualizar_postura/1 -> Conversion boolean a int8 2/1
  Generic Shared Memory Block V2.0/1 -> "Conversion Float a Doble "/1
  Conversion Doble a Float1/1 -> Generic Shared Memory Block V2.0/2

== system LibrarySimulinkSASv2016a/V-REP Mobile Manipulator V3/V-REP Manipulator ==
  Joints Target Velocity : Inport
  Posture : Inport  Port=2
  Gripper Close : Inport  Port=3
  Target Position/Orientation : Inport  Port=4
  Conversion Doble a Float : DataTypeConversion  OutDataTypeStr=single
  Conversion Doble a Float1 : DataTypeConversion  OutDataTypeStr=single
  Conversion Doble a Float2 : DataTypeConversion  OutDataTypeStr=single
  Conversion Doble a Float3 : DataTypeConversion  OutDataTypeStr=int32
  "Conversion Float a Doble " : DataTypeConversion
  Conversion Float a Doble 2 : DataTypeConversion
  Conversion Float a Doble 3 : DataTypeConversion
  Generic Shared Memory Block V2.0 : S-Function  FunctionName=matlabGenericSHM  Parameters=nombre,dispositivo,vecE,vecS,tipoE,tipoS  SFunctionDeploymentMode=off  EnableBusSupport=off  mask(Generic Share Memory block V2.0){nombre='RoboArm', dispositivo=id, vecE=[4 4 1 6], vecS=[6 4 4], tipoE=['f' 'f' 'i' 'f'], tipoS=['f' 'f' 'f']}
      mask prompts: nombre "Share Memory base name"; dispositivo "Device Number"; vecE "Sizes input vector"; vecS "Sizes output vector"; tipoE "Data Types Inputs"; tipoS "Data Types Outputs"
      mask help:
      | This block allows Simulink communication with external processes through shared memory. It works on Unix and Windows.
      | More information in Help.
      mask Help:
      | Share Memory base name (nombre): It's the base string of share memory ej 'robot'
      | Device Number (dispositivo): integer that identifies the device between multiple in use
      | Two shared memorys are created with names:
      | "%name_entrada_%dispositivo" for the device input (writing)
      | "%name_salida_%dispositivo" for the device output (reading)
      | Sizes input vector (vecE)  : array that specify the number of inputs (array dimension) and the inputs size (array values)
      | Sizes output vector  (vecS): array that specify the number of outputs (array dimension) and the outputs size (array values)
      | Data types input(tipoE): char array that specify each input types
      | Data types output (tipoS): char array that specify each output types
      | Allowed types are:
      | ('f'=float, 'd'=double, 'i'=int32, 'c'=char, 'C'= unsigned char, 'I'=unsigned int)
      | Example.
      | Suppose the following parameters:
      | nombre='exampleMemory'
      | dispositivo=10
      | vecE=[3 2 1]
      | vecS=[6 5 7 8]
      | tipoE=['f' 'I' 'c']
      | tipoS=['C' 'i' 'd' 'd']
      | 
      | Two Share Memories are created with names:
      | "exampleMemory_entrada_10" (block input)
      | "exampleMemory_salida_10"  (block output)
      | 3 inputs are created:
      |  1° Dimension 3, type "unsigned char"
      |  2° Dimension 2, type "int"
      |  3° Dimension 1, type "char"
      | 4 outputs are created:
      |  1° Dimension 6, type "float"
      |  2° Dimension 5, type "unsigned int"
      |  3° Dimension 7, type "double"
      |  3° Dimension 8, type "double"
      mask Display: image('shmem.jpg')
  Tip Position : Outport
  Joints State : Outport  Port=2
  Joints Velocity : Outport  Port=3
  wires:
  Conversion Doble a Float3/1 -> Generic Shared Memory Block V2.0/3
  Generic Shared Memory Block V2.0/2 -> Conversion Float a Doble 2/1
  Generic Shared Memory Block V2.0/3 -> Conversion Float a Doble 3/1
  Conversion Float a Doble 3/1 -> Joints Velocity/1
  "Conversion Float a Doble "/1 -> Tip Position/1
  Conversion Float a Doble 2/1 -> Joints State/1
  Generic Shared Memory Block V2.0/1 -> "Conversion Float a Doble "/1
  Joints Target Velocity/1 -> Conversion Doble a Float/1
  Gripper Close/1 -> Conversion Doble a Float3/1
  Target Position/Orientation/1 -> Conversion Doble a Float1/1
  Conversion Doble a Float/1 -> Generic Shared Memory Block V2.0/1
  Conversion Doble a Float1/1 -> Generic Shared Memory Block V2.0/4
  Posture/1 -> Conversion Doble a Float2/1
  Conversion Doble a Float2/1 -> Generic Shared Memory Block V2.0/2

== matlab function LibrarySimulinkSASv2016a/plantilla IApp/MATLAB Function ==
  data: appControl input; lastControl input; y output
function y = fcn(appControl,lastControl)
if(appControl~=lastControl)
    y=1;
else
    y=0;
end

== matlab function LibrarySimulinkSASv2016a/ISharedMemory Control/MATLAB Function3 ==
  data: u input; y output
function y = fcn(u)
y=zeros(40,1);
y(1:length(u),1)=u;

== matlab function LibrarySimulinkSASv2016a/UDP Data Client/MATLAB Function ==
  data: data input; size output; array output
function [size,array] = fillData(data)
n=length(data);
size=uint32(n);
array = [data;zeros(1024-n,1)];

== matlab function LibrarySimulinkSASv2016a/plantilla IApp/sizeCalc ==
  data: size input; y output; type input
function y = fcn(size,type)
n=length(size);
y=0;
for i=1:n
    switch type(i)
        case 'c'
            aux=size(i)*1;
        case 'C'
            aux=size(i)*1;
        case 'd'
            aux=size(i)*6;
        case 'f'
            aux=size(i)*4;
        case 'i'
            aux=size(i)*4;
        case 'I'
            aux=size(i)*4;
        otherwise %double
            aux=size(i)*6;
    end
y=y+aux;
end
end
